simulink model "HvacCtrlrAirMFlowFrnt"  (R2022b)
settings: Solver=FixedStepDiscrete  StartTime=0.0  StopTime=10.0  FixedStep=0.2  RelTol=1e-3
(part 2 of 2)

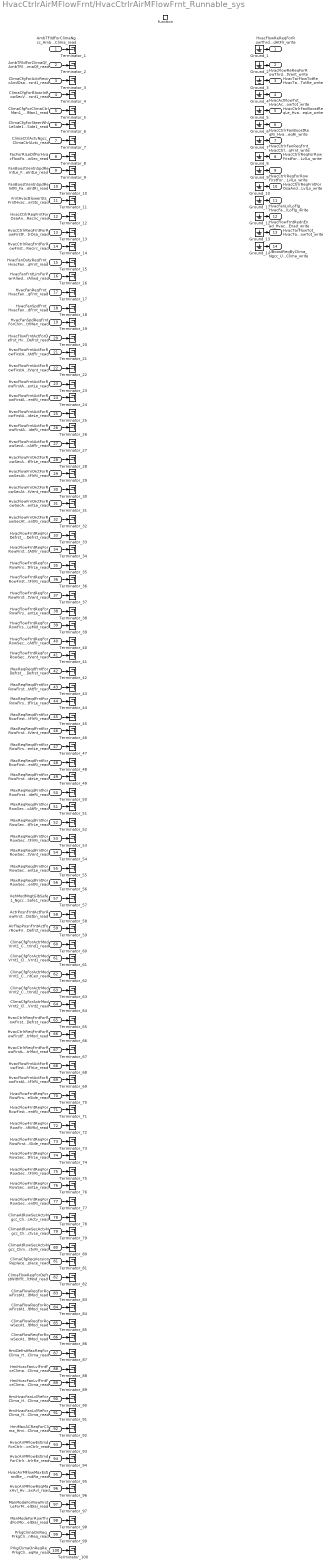
== system HvacCtrlrAirMFlowFrnt/HvacCtrlrAirMFlowFrnt_Runnable_sys ==
  AmbTFildForClimaNgcc_AmbTFildForClima_read : Inport
  AmbTFildForClimaQf_AmbTFildForClimaQf_read : Inport  Port=2
  ClimaCfgForActrRecircAndOsaCmnPrsnt1_ClimaCfgForActrRecircAndOsaCmnPrsnt1_read : Inport  Port=3
  ClimaCfgForBlowrInRowSecVentSidePrsnt1_ClimaCfgForBlowrInRowSecVentSidePrsnt1_read : Inport  Port=4
  ClimaCfgForClimaCtrlMan1_ClimaCfgForClimaCtrlMan1_read : Inport  Port=5
  ClimaCfgForSteerWhlLeSide1_ClimaCfgForSteerWhlLeSide1_read : Inport  Port=6
  ClimaCtrlActvNgcc_ClimaCtrlActv_read : Inport  Port=7
  FacForRiLeDifForHvacFlowForVentRowSec_FacForRiLeDifForHvacFlowForVentRowSec_read : Inport  Port=8
  FanBoostVentnSpdReIntLe_FanBoostVentnSpdReIntLe_read : Inport  Port=9
  FanBoostVentnSpdReIntRi_FanBoostVentnSpdReIntRi_read : Inport  Port=10
  FrntHvacBlowerSts_FrntHvacBlowerSts_read : Inport  Port=11
  HvacCtrlrReqFrntForOsaAndRecirc_HvacCtrlrReqFrntForOsaAndRecirc_read : Inport  Port=12
  HvacCtrlrReqFrntForRowFirstForActrOsa_HvacCtrlrReqFrntForRowFirstForActrOsa_read : Inport  Port=13
  HvacCtrlrReqFrntForRowFirstForActrRecirc_HvacCtrlrReqFrntForRowFirstForActrRecirc_read : Inport  Port=14
  HvacFanDutyReqFrnt_HvacFanDutyReqFrnt_read : Inport  Port=15
  HvacFanFrntLimForPwrAllwd_HvacFanFrntLimForPwrAllwd_read : Inport  Port=16
  HvacFanReqFrnt_HvacFanReqFrnt_read : Inport  Port=17
  HvacFanSpdFrnt_HvacFanSpdFrnt_read : Inport  Port=18
  HvacFanSpdReqFrntForClimaCtrlMan_HvacFanSpdReqFrntForClimaCtrlMan_read : Inport  Port=19
  HvacFlowFrntActForDefrst_HvacFlowFrntActForDefrst_read : Inport  Port=20
  HvacFlowFrntActForRowFirstAtFlr_HvacFlowFrntActForRowFirstAtFlr_read : Inport  Port=21
  HvacFlowFrntActForRowFirstAtVent_HvacFlowFrntActForRowFirstAtVent_read : Inport  Port=22
  HvacFlowFrntActForRowFirstAtVent_HvacFlowFrntActForRowFirstAtVentLe_read : Inport  Port=23
  HvacFlowFrntActForRowFirstAtVent_HvacFlowFrntActForRowFirstAtVentRi_read : Inport  Port=24
  HvacFlowFrntActForRowFirstAtVent_HvacFlowFrntActForRowFirstAtVentSideLe_read : Inport  Port=25
  HvacFlowFrntActForRowFirstAtVent_HvacFlowFrntActForRowFirstAtVentSideRi_read : Inport  Port=26
  HvacFlowFrntActForRowSecAtFlr_HvacFlowFrntActForRowSecAtFlr_read : Inport  Port=27
  HvacFlowFrntActForRowSecAtFlr_HvacFlowFrntActForRowSecAtFlrLe_read : Inport  Port=28
  HvacFlowFrntActForRowSecAtFlr_HvacFlowFrntActForRowSecAtFlrRi_read : Inport  Port=29
  HvacFlowFrntActForRowSecAtVent_HvacFlowFrntActForRowSecAtVent_read : Inport  Port=30
  HvacFlowFrntActForRowSecAtVent_HvacFlowFrntActForRowSecAtVentLe_read : Inport  Port=31
  HvacFlowFrntActForRowSecAtVent_HvacFlowFrntActForRowSecAtVentRi_read : Inport  Port=32
  HvacFlowFrntReqForDefrst_HvacFlowFrntReqForDefrst_read : Inport  Port=33
  HvacFlowFrntReqForRowFirstAtFlr_HvacFlowFrntReqForRowFirstAtFlr_read : Inport  Port=34
  HvacFlowFrntReqForRowFirstAtFlr_HvacFlowFrntReqForRowFirstAtFlrLe_read : Inport  Port=35
  HvacFlowFrntReqForRowFirstAtFlr_HvacFlowFrntReqForRowFirstAtFlrRi_read : Inport  Port=36
  HvacFlowFrntReqForRowFirstAtVent_HvacFlowFrntReqForRowFirstAtVent_read : Inport  Port=37
  HvacFlowFrntReqForRowFirstAtVent_HvacFlowFrntReqForRowFirstAtVentLe_read : Inport  Port=38
  HvacFlowFrntReqForRowFirstAtVent_HvacFlowFrntReqForRowFirstAtVentLeMid_read : Inport  Port=39
  HvacFlowFrntReqForRowSecAtFlr_HvacFlowFrntReqForRowSecAtFlr_read : Inport  Port=40
  HvacFlowFrntReqForRowSecAtVent_HvacFlowFrntReqForRowSecAtVent_read : Inport  Port=41
  MaxReqReqdFrntForDefrst_MaxReqReqdFrntForDefrst_read : Inport  Port=42
  MaxReqReqdFrntForRowFirstAtFlr_MaxReqReqdFrntForRowFirstAtFlr_read : Inport  Port=43
  MaxReqReqdFrntForRowFirstAtFlr_MaxReqReqdFrntForRowFirstAtFlrLe_read : Inport  Port=44
  MaxReqReqdFrntForRowFirstAtFlr_MaxReqReqdFrntForRowFirstAtFlrRi_read : Inport  Port=45
  MaxReqReqdFrntForRowFirstAtVent_MaxReqReqdFrntForRowFirstAtVent_read : Inport  Port=46
  MaxReqReqdFrntForRowFirstAtVent_MaxReqReqdFrntForRowFirstAtVentLe_read : Inport  Port=47
  MaxReqReqdFrntForRowFirstAtVent_MaxReqReqdFrntForRowFirstAtVentRi_read : Inport  Port=48
  MaxReqReqdFrntForRowFirstAtVent_MaxReqReqdFrntForRowFirstAtVentSideLe_read : Inport  Port=49
  MaxReqReqdFrntForRowFirstAtVent_MaxReqReqdFrntForRowFirstAtVentSideRi_read : Inport  Port=50
  MaxReqReqdFrntForRowSecAtFlr_MaxReqReqdFrntForRowSecAtFlr_read : Inport  Port=51
  MaxReqReqdFrntForRowSecAtFlr_MaxReqReqdFrntForRowSecAtFlrLe_read : Inport  Port=52
  MaxReqReqdFrntForRowSecAtFlr_MaxReqReqdFrntForRowSecAtFlrRi_read : Inport  Port=53
  MaxReqReqdFrntForRowSecAtVent_MaxReqReqdFrntForRowSecAtVent_read : Inport  Port=54
  MaxReqReqdFrntForRowSecAtVent_MaxReqReqdFrntForRowSecAtVentLe_read : Inport  Port=55
  MaxReqReqdFrntForRowSecAtVent_MaxReqReqdFrntForRowSecAtVentRi_read : Inport  Port=56
  VehModMngtGlbSafe1_Ngcc_VehModMngtGlbSafe1_read : Inport  Port=57
  ActrPosnFrntActForRowFirstModDistbn_ActrPosnFrntActForRowFirstModDistbn_read : Inport  Port=58
  AirFlapPosnFrntActForRowFirstForActrDefrst_AirFlapPosnFrntActForRowFirstForActrDefrst_read : Inport  Port=59
  ClimaCfgForActrModVrnt1_ClimaCfgForActrInd1_read : Inport  Port=60
  ClimaCfgForActrModVrnt1_ClimaCfgForActrModVrnt1_read : Inport  Port=61
  ClimaCfgForActrModVrnt1_ClimaCfgForActrModVrntCen_read : Inport  Port=62
  ClimaCfgForActrModVrnt2_ClimaCfgForActrInd2_read : Inport  Port=63
  ClimaCfgForActrModVrnt2_ClimaCfgForActrModVrnt2_read : Inport  Port=64
  HvacCtrlrReqFrntForRowFirstForActrDefrst_HvacCtrlrReqFrntForRowFirstForActrDefrst_read : Inport  Port=65
  HvacCtrlrReqFrntForRowFirstForActrMod_HvacCtrlrReqFrntForRowFirstForActrMod_read : Inport  Port=66
  HvacCtrlrReqFrntForRowFirstLeForActrMod_HvacCtrlrReqFrntForRowFirstLeForActrMod_read : Inport  Port=67
  HvacFlowFrntActForRowFirstAtFlr_HvacFlowFrntActForRowFirstAtFlrLe_read : Inport  Port=68
  HvacFlowFrntActForRowFirstAtFlr_HvacFlowFrntActForRowFirstAtFlrRi_read : Inport  Port=69
  HvacFlowFrntReqForRowFirstAtVent_HvacFlowFrntReqForRowFirstAtVentLeSide_read : Inport  Port=70
  HvacFlowFrntReqForRowFirstAtVent_HvacFlowFrntReqForRowFirstAtVentRi_read : Inport  Port=71
  HvacFlowFrntReqForRowFirstAtVent_HvacFlowFrntReqForRowFirstAtVentRiMid_read : Inport  Port=72
  HvacFlowFrntReqForRowFirstAtVent_HvacFlowFrntReqForRowFirstAtVentRiSide_read : Inport  Port=73
  HvacFlowFrntReqForRowSecAtFlr_HvacFlowFrntReqForRowSecAtFlrLe_read : Inport  Port=74
  HvacFlowFrntReqForRowSecAtFlr_HvacFlowFrntReqForRowSecAtFlrRi_read : Inport  Port=75
  HvacFlowFrntReqForRowSecAtVent_HvacFlowFrntReqForRowSecAtVentLe_read : Inport  Port=76
  HvacFlowFrntReqForRowSecAtVent_HvacFlowFrntReqForRowSecAtVentRi_read : Inport  Port=77
  ClimaAtRowSecActvNgcc_ClimaAtRowSecActv_read : Inport  Port=78
  ClimaAtRowSecActvNgcc_ClimaAtRowSecActvLe_read : Inport  Port=79
  ClimaAtRowSecActvNgcc_ClimaAtRowSecActvRi_read : Inport  Port=80
  ClimaCfgReqVersionReplace_ClimaCfgReqVersionReplace_read : Inport  Port=81
  ClimaFlowReqForDefrstWithFltMod_ClimaFlowReqForDefrstWithFltMod_read : Inport  Port=82
  ClimaFlowReqForRowFirstAtFlrWithFltMod_ClimaFlowReqForRowFirstAtFlrWithFltMod_read : Inport  Port=83
  ClimaFlowReqForRowFirstAtVentWithFltMod_ClimaFlowReqForRowFirstAtVentWithFltMod_read : Inport  Port=84
  ClimaFlowReqForRowSecAtFlrWithFltMod_ClimaFlowReqForRowSecAtFlrWithFltMod_read : Inport  Port=85
  ClimaFlowReqForRowSecAtVentWithFltMod_ClimaFlowReqForRowSecAtVentWithFltMod_read : Inport  Port=86
  HmiDefrstMaxReqForClima_HmiDefrstMaxReqForClima_read : Inport  Port=87
  HmiHvacFanLvlFrntForClima_HmiHvacFanLvlFrntForClima_read : Inport  Port=88
  HmiHvacFanLvlFrntForClima_HmiHvacFanLvlFrntRiForClima_read : Inport  Port=89
  HmiHvacFanLvlReForClima_HmiHvacFanLvlReForClima_read : Inport  Port=90
  HmiHvacFanLvlReForClima_HmiHvacFanLvlReRiForClima_read : Inport  Port=91
  HmiMaxACReqForClima_HmiMaxACReqForClima_read : Inport  Port=92
  HvacAirMFlowEstimdForCtrlr_HvacAirMFlowEstimdForCtrlr_read : Inport  Port=93
  HvacAirMFlowEstimdForCtrlrRe_HvacAirMFlowEstimdForCtrlrRe_read : Inport  Port=94
  HvacAirMFlowMaxEstimdRe_HvacAirMFlowMaxEstimdRe_read : Inport  Port=95
  HvacAirMFlowReqMaxAvl_HvacAirMFlowReqMaxAvl_read : Inport  Port=96
  ManModeForRowFirstLeForModelDial_ManModeForRowFirstLeForModelDial_read : Inport  Port=97
  ManModeForRowThrdForModelDial_ManModeForRowThrdForModelDial_read : Inport  Port=98
  PrkgClimaOnReq_PrkgClimaOnReq_read : Inport  Port=99
  PrkgClimaOnReqRe_PrkgClimaOnReqRe_read : Inport  Port=100
  function : TriggerPort  TriggerType=function-call  VariantControl=(inherit)  SampleTimeType=periodic  SampleTime=-1
  Ground_{1..14} (14 alike) : Ground
  Terminator_{1..100} (100 alike) : Terminator
  HvacFlowReReqForRowThrdAtFlr_HvacFlowReReqForRowThrdAtFlr_write : Outport
      settings: EnsureOutportIsVirtual=on
  HvacFlowReReqForRowThrdAtVent_HvacFlowReReqForRowThrdAtVent_write : Outport  Port=2
      settings: EnsureOutportIsVirtual=on
  HvacTarFlowTotRe_HvacTarFlowTotRe_write : Outport  Port=3
      settings: EnsureOutportIsVirtual=on
  HvacActFlowTot_HvacActFlowTot_write : Outport  Port=4
      settings: EnsureOutportIsVirtual=on
  HvacCtrlrFanBoostReqLe_HvacCtrlrFanBoostReqLe_write : Outport  Port=5
      settings: EnsureOutportIsVirtual=on
  HvacCtrlrFanBoostReqRi_HvacCtrlrFanBoostReqRi_write : Outport  Port=6
      settings: EnsureOutportIsVirtual=on
  HvacCtrlrFanReqFrnt_HvacCtrlrFanReqFrnt_write : Outport  Port=7
      settings: EnsureOutportIsVirtual=on
  HvacCtrlrReqForRowFirstForActrOsaForFanLvlLo_HvacCtrlrReqForRowFirstForActrOsaForFanLvlLo_write : Outport  Port=8
      settings: EnsureOutportIsVirtual=on
  HvacCtrlrReqForRowFirstForActrRecircForFanLvlLo_HvacCtrlrReqForRowFirstForActrRecircForFanLvlLo_write : Outport  Port=9
      settings: EnsureOutportIsVirtual=on
  HvacCtrlrReqFrntForOsaAndRecircForFanLvlLo_HvacCtrlrReqFrntForOsaAndRecircForFanLvlLo_write : Outport  Port=10
      settings: EnsureOutportIsVirtual=on
  HvacFanLvlLoFlg_HvacFanLvlLoFlg_write : Outport  Port=11
      settings: EnsureOutportIsVirtual=on
  HvacFlowFrntRednEnad_HvacFlowFrntRednEnad_write : Outport  Port=12
      settings: EnsureOutportIsVirtual=on
  HvacTarFlowTot_HvacTarFlowTot_write : Outport  Port=13
      settings: EnsureOutportIsVirtual=on
  UBoostReqByClima_Ngcc_UBoostReqByClima_write : Outport  Port=14
      settings: EnsureOutportIsVirtual=on
  wires:
  AmbTFildForClimaNgcc_AmbTFildForClima_read/1 -> Terminator_1/1
  AmbTFildForClimaQf_AmbTFildForClimaQf_read/1 -> Terminator_2/1
  ClimaCfgForActrRecircAndOsaCmnPrsnt1_ClimaCfgForActrRecircAndOsaCmnPrsnt1_read/1 -> Terminator_3/1
  ClimaCfgForBlowrInRowSecVentSidePrsnt1_ClimaCfgForBlowrInRowSecVentSidePrsnt1_read/1 -> Terminator_4/1
  ClimaCfgForClimaCtrlMan1_ClimaCfgForClimaCtrlMan1_read/1 -> Terminator_5/1
  ClimaCfgForSteerWhlLeSide1_ClimaCfgForSteerWhlLeSide1_read/1 -> Terminator_6/1
  ClimaCtrlActvNgcc_ClimaCtrlActv_read/1 -> Terminator_7/1
  FacForRiLeDifForHvacFlowForVentRowSec_FacForRiLeDifForHvacFlowForVentRowSec_read/1 -> Terminator_8/1
  FanBoostVentnSpdReIntLe_FanBoostVentnSpdReIntLe_read/1 -> Terminator_9/1
  FanBoostVentnSpdReIntRi_FanBoostVentnSpdReIntRi_read/1 -> Terminator_10/1
  FrntHvacBlowerSts_FrntHvacBlowerSts_read/1 -> Terminator_11/1
  HvacCtrlrReqFrntForOsaAndRecirc_HvacCtrlrReqFrntForOsaAndRecirc_read/1 -> Terminator_12/1
  HvacCtrlrReqFrntForRowFirstForActrOsa_HvacCtrlrReqFrntForRowFirstForActrOsa_read/1 -> Terminator_13/1
  HvacCtrlrReqFrntForRowFirstForActrRecirc_HvacCtrlrReqFrntForRowFirstForActrRecirc_read/1 -> Terminator_14/1
  HvacFanDutyReqFrnt_HvacFanDutyReqFrnt_read/1 -> Terminator_15/1
  HvacFanFrntLimForPwrAllwd_HvacFanFrntLimForPwrAllwd_read/1 -> Terminator_16/1
  HvacFanReqFrnt_HvacFanReqFrnt_read/1 -> Terminator_17/1
  HvacFanSpdFrnt_HvacFanSpdFrnt_read/1 -> Terminator_18/1
  HvacFanSpdReqFrntForClimaCtrlMan_HvacFanSpdReqFrntForClimaCtrlMan_read/1 -> Terminator_19/1
  HvacFlowFrntActForDefrst_HvacFlowFrntActForDefrst_read/1 -> Terminator_20/1
  HvacFlowFrntActForRowFirstAtFlr_HvacFlowFrntActForRowFirstAtFlr_read/1 -> Terminator_21/1
  HvacFlowFrntActForRowFirstAtVent_HvacFlowFrntActForRowFirstAtVent_read/1 -> Terminator_22/1
  HvacFlowFrntActForRowFirstAtVent_HvacFlowFrntActForRowFirstAtVentLe_read/1 -> Terminator_23/1
  HvacFlowFrntActForRowFirstAtVent_HvacFlowFrntActForRowFirstAtVentRi_read/1 -> Terminator_24/1
  HvacFlowFrntActForRowFirstAtVent_HvacFlowFrntActForRowFirstAtVentSideLe_read/1 -> Terminator_25/1
  HvacFlowFrntActForRowFirstAtVent_HvacFlowFrntActForRowFirstAtVentSideRi_read/1 -> Terminator_26/1
  HvacFlowFrntActForRowSecAtFlr_HvacFlowFrntActForRowSecAtFlr_read/1 -> Terminator_27/1
  HvacFlowFrntActForRowSecAtFlr_HvacFlowFrntActForRowSecAtFlrLe_read/1 -> Terminator_28/1
  HvacFlowFrntActForRowSecAtFlr_HvacFlowFrntActForRowSecAtFlrRi_read/1 -> Terminator_29/1
  HvacFlowFrntActForRowSecAtVent_HvacFlowFrntActForRowSecAtVent_read/1 -> Terminator_30/1
  HvacFlowFrntActForRowSecAtVent_HvacFlowFrntActForRowSecAtVentLe_read/1 -> Terminator_31/1
  HvacFlowFrntActForRowSecAtVent_HvacFlowFrntActForRowSecAtVentRi_read/1 -> Terminator_32/1
  HvacFlowFrntReqForDefrst_HvacFlowFrntReqForDefrst_read/1 -> Terminator_33/1
  HvacFlowFrntReqForRowFirstAtFlr_HvacFlowFrntReqForRowFirstAtFlr_read/1 -> Terminator_34/1
  HvacFlowFrntReqForRowFirstAtFlr_HvacFlowFrntReqForRowFirstAtFlrLe_read/1 -> Terminator_35/1
  HvacFlowFrntReqForRowFirstAtFlr_HvacFlowFrntReqForRowFirstAtFlrRi_read/1 -> Terminator_36/1
  HvacFlowFrntReqForRowFirstAtVent_HvacFlowFrntReqForRowFirstAtVent_read/1 -> Terminator_37/1
  HvacFlowFrntReqForRowFirstAtVent_HvacFlowFrntReqForRowFirstAtVentLe_read/1 -> Terminator_38/1
  HvacFlowFrntReqForRowFirstAtVent_HvacFlowFrntReqForRowFirstAtVentLeMid_read/1 -> Terminator_39/1
  HvacFlowFrntReqForRowSecAtFlr_HvacFlowFrntReqForRowSecAtFlr_read/1 -> Terminator_40/1
  HvacFlowFrntReqForRowSecAtVent_HvacFlowFrntReqForRowSecAtVent_read/1 -> Terminator_41/1
  MaxReqReqdFrntForDefrst_MaxReqReqdFrntForDefrst_read/1 -> Terminator_42/1
  MaxReqReqdFrntForRowFirstAtFlr_MaxReqReqdFrntForRowFirstAtFlr_read/1 -> Terminator_43/1
  MaxReqReqdFrntForRowFirstAtFlr_MaxReqReqdFrntForRowFirstAtFlrLe_read/1 -> Terminator_44/1
  MaxReqReqdFrntForRowFirstAtFlr_MaxReqReqdFrntForRowFirstAtFlrRi_read/1 -> Terminator_45/1
  MaxReqReqdFrntForRowFirstAtVent_MaxReqReqdFrntForRowFirstAtVent_read/1 -> Terminator_46/1
  MaxReqReqdFrntForRowFirstAtVent_MaxReqReqdFrntForRowFirstAtVentLe_read/1 -> Terminator_47/1
  MaxReqReqdFrntForRowFirstAtVent_MaxReqReqdFrntForRowFirstAtVentRi_read/1 -> Terminator_48/1
  MaxReqReqdFrntForRowFirstAtVent_MaxReqReqdFrntForRowFirstAtVentSideLe_read/1 -> Terminator_49/1
  MaxReqReqdFrntForRowFirstAtVent_MaxReqReqdFrntForRowFirstAtVentSideRi_read/1 -> Terminator_50/1
  MaxReqReqdFrntForRowSecAtFlr_MaxReqReqdFrntForRowSecAtFlr_read/1 -> Terminator_51/1
  MaxReqReqdFrntForRowSecAtFlr_MaxReqReqdFrntForRowSecAtFlrLe_read/1 -> Terminator_52/1
  MaxReqReqdFrntForRowSecAtFlr_MaxReqReqdFrntForRowSecAtFlrRi_read/1 -> Terminator_53/1
  MaxReqReqdFrntForRowSecAtVent_MaxReqReqdFrntForRowSecAtVent_read/1 -> Terminator_54/1
  MaxReqReqdFrntForRowSecAtVent_MaxReqReqdFrntForRowSecAtVentLe_read/1 -> Terminator_55/1
  MaxReqReqdFrntForRowSecAtVent_MaxReqReqdFrntForRowSecAtVentRi_read/1 -> Terminator_56/1
  VehModMngtGlbSafe1_Ngcc_VehModMngtGlbSafe1_read/1 -> Terminator_57/1
  ActrPosnFrntActForRowFirstModDistbn_ActrPosnFrntActForRowFirstModDistbn_read/1 -> Terminator_58/1
  AirFlapPosnFrntActForRowFirstForActrDefrst_AirFlapPosnFrntActForRowFirstForActrDefrst_read/1 -> Terminator_59/1
  ClimaCfgForActrModVrnt1_ClimaCfgForActrInd1_read/1 -> Terminator_60/1
  ClimaCfgForActrModVrnt1_ClimaCfgForActrModVrnt1_read/1 -> Terminator_61/1
  ClimaCfgForActrModVrnt1_ClimaCfgForActrModVrntCen_read/1 -> Terminator_62/1
  ClimaCfgForActrModVrnt2_ClimaCfgForActrInd2_read/1 -> Terminator_63/1
  ClimaCfgForActrModVrnt2_ClimaCfgForActrModVrnt2_read/1 -> Terminator_64/1
  HvacCtrlrReqFrntForRowFirstForActrDefrst_HvacCtrlrReqFrntForRowFirstForActrDefrst_read/1 -> Terminator_65/1
  HvacCtrlrReqFrntForRowFirstForActrMod_HvacCtrlrReqFrntForRowFirstForActrMod_read/1 -> Terminator_66/1
  HvacCtrlrReqFrntForRowFirstLeForActrMod_HvacCtrlrReqFrntForRowFirstLeForActrMod_read/1 -> Terminator_67/1
  HvacFlowFrntActForRowFirstAtFlr_HvacFlowFrntActForRowFirstAtFlrLe_read/1 -> Terminator_68/1
  HvacFlowFrntActForRowFirstAtFlr_HvacFlowFrntActForRowFirstAtFlrRi_read/1 -> Terminator_69/1
  HvacFlowFrntReqForRowFirstAtVent_HvacFlowFrntReqForRowFirstAtVentLeSide_read/1 -> Terminator_70/1
  HvacFlowFrntReqForRowFirstAtVent_HvacFlowFrntReqForRowFirstAtVentRi_read/1 -> Terminator_71/1
  HvacFlowFrntReqForRowFirstAtVent_HvacFlowFrntReqForRowFirstAtVentRiMid_read/1 -> Terminator_72/1
  HvacFlowFrntReqForRowFirstAtVent_HvacFlowFrntReqForRowFirstAtVentRiSide_read/1 -> Terminator_73/1
  HvacFlowFrntReqForRowSecAtFlr_HvacFlowFrntReqForRowSecAtFlrLe_read/1 -> Terminator_74/1
  HvacFlowFrntReqForRowSecAtFlr_HvacFlowFrntReqForRowSecAtFlrRi_read/1 -> Terminator_75/1
  HvacFlowFrntReqForRowSecAtVent_HvacFlowFrntReqForRowSecAtVentLe_read/1 -> Terminator_76/1
  HvacFlowFrntReqForRowSecAtVent_HvacFlowFrntReqForRowSecAtVentRi_read/1 -> Terminator_77/1
  ClimaAtRowSecActvNgcc_ClimaAtRowSecActv_read/1 -> Terminator_78/1
  ClimaAtRowSecActvNgcc_ClimaAtRowSecActvLe_read/1 -> Terminator_79/1
  ClimaAtRowSecActvNgcc_ClimaAtRowSecActvRi_read/1 -> Terminator_80/1
  ClimaCfgReqVersionReplace_ClimaCfgReqVersionReplace_read/1 -> Terminator_81/1
  ClimaFlowReqForDefrstWithFltMod_ClimaFlowReqForDefrstWithFltMod_read/1 -> Terminator_82/1
  ClimaFlowReqForRowFirstAtFlrWithFltMod_ClimaFlowReqForRowFirstAtFlrWithFltMod_read/1 -> Terminator_83/1
  ClimaFlowReqForRowFirstAtVentWithFltMod_ClimaFlowReqForRowFirstAtVentWithFltMod_read/1 -> Terminator_84/1
  ClimaFlowReqForRowSecAtFlrWithFltMod_ClimaFlowReqForRowSecAtFlrWithFltMod_read/1 -> Terminator_85/1
  ClimaFlowReqForRowSecAtVentWithFltMod_ClimaFlowReqForRowSecAtVentWithFltMod_read/1 -> Terminator_86/1
  HmiDefrstMaxReqForClima_HmiDefrstMaxReqForClima_read/1 -> Terminator_87/1
  HmiHvacFanLvlFrntForClima_HmiHvacFanLvlFrntForClima_read/1 -> Terminator_88/1
  HmiHvacFanLvlFrntForClima_HmiHvacFanLvlFrntRiForClima_read/1 -> Terminator_89/1
  HmiHvacFanLvlReForClima_HmiHvacFanLvlReForClima_read/1 -> Terminator_90/1
  HmiHvacFanLvlReForClima_HmiHvacFanLvlReRiForClima_read/1 -> Terminator_91/1
  HmiMaxACReqForClima_HmiMaxACReqForClima_read/1 -> Terminator_92/1
  HvacAirMFlowEstimdForCtrlr_HvacAirMFlowEstimdForCtrlr_read/1 -> Terminator_93/1
  HvacAirMFlowEstimdForCtrlrRe_HvacAirMFlowEstimdForCtrlrRe_read/1 -> Terminator_94/1
  HvacAirMFlowMaxEstimdRe_HvacAirMFlowMaxEstimdRe_read/1 -> Terminator_95/1
  HvacAirMFlowReqMaxAvl_HvacAirMFlowReqMaxAvl_read/1 -> Terminator_96/1
  ManModeForRowFirstLeForModelDial_ManModeForRowFirstLeForModelDial_read/1 -> Terminator_97/1
  ManModeForRowThrdForModelDial_ManModeForRowThrdForModelDial_read/1 -> Terminator_98/1
  PrkgClimaOnReq_PrkgClimaOnReq_read/1 -> Terminator_99/1
  PrkgClimaOnReqRe_PrkgClimaOnReqRe_read/1 -> Terminator_100/1
  Ground_1/1 -> HvacFlowReReqForRowThrdAtFlr_HvacFlowReReqForRowThrdAtFlr_write/1
  Ground_2/1 -> HvacFlowReReqForRowThrdAtVent_HvacFlowReReqForRowThrdAtVent_write/1
  Ground_3/1 -> HvacTarFlowTotRe_HvacTarFlowTotRe_write/1
  Ground_4/1 -> HvacActFlowTot_HvacActFlowTot_write/1
  Ground_5/1 -> HvacCtrlrFanBoostReqLe_HvacCtrlrFanBoostReqLe_write/1
  Ground_6/1 -> HvacCtrlrFanBoostReqRi_HvacCtrlrFanBoostReqRi_write/1
  Ground_7/1 -> HvacCtrlrFanReqFrnt_HvacCtrlrFanReqFrnt_write/1
  Ground_8/1 -> HvacCtrlrReqForRowFirstForActrOsaForFanLvlLo_HvacCtrlrReqForRowFirstForActrOsaForFanLvlLo_write/1
  Ground_9/1 -> HvacCtrlrReqForRowFirstForActrRecircForFanLvlLo_HvacCtrlrReqForRowFirstForActrRecircForFanLvlLo_write/1
  Ground_10/1 -> HvacCtrlrReqFrntForOsaAndRecircForFanLvlLo_HvacCtrlrReqFrntForOsaAndRecircForFanLvlLo_write/1
  Ground_11/1 -> HvacFanLvlLoFlg_HvacFanLvlLoFlg_write/1
  Ground_12/1 -> HvacFlowFrntRednEnad_HvacFlowFrntRednEnad_write/1
  Ground_13/1 -> HvacTarFlowTot_HvacTarFlowTot_write/1
  Ground_14/1 -> UBoostReqByClima_Ngcc_UBoostReqByClima_write/1
